simulink model "vegas01_4tap_1024ch_220_r7b"  (Simulink 7.6)
settings: Solver=ode45  StartTime=0.0  StopTime=128000  RelTol=1e-3
libraries: xbsIndex_r4 x13489, monroe_library x80, xps_library x51, casper_library_misc x43, xbsReplacements_r4 x42, dspsigops x16, simulink x7
(part 13 of 99)

== system vegas01_4tap_1024ch_220_r7b/pfb_fir_real3/coeff_gen_3_4 ==
  sync : Inport
  Counter0 : xbsIndex_r4/Counter  cnt_type=Free Running  cnt_to=Inf  operation=Up  start_count=8  cnt_by_val=1  arith_type=Unsigned  n_bits=8  bin_pt=0  load_pin=off  rst=on  en=off  explicit_period=on  period=1  dbl_ovrd=off  use_behavioral_HDL=off  implementation=Fabric  block_type=counter
  Counter1 : xbsIndex_r4/Counter  cnt_type=Free Running  cnt_to=Inf  operation=Down  start_count=247  cnt_by_val=1  arith_type=Unsigned  n_bits=8  bin_pt=0  load_pin=off  rst=on  en=off  explicit_period=on  period=1  dbl_ovrd=off  use_behavioral_HDL=off  implementation=Fabric  block_type=counter
  Delay16 : xbsIndex_r4/Delay  rst=off  infoeditControl=Selection of Reset will increase slice count due to use of real FFs and instead of SRLs  en=off  latency=2  dbl_ovrd=off  reg_retiming=off  block_type=delay
  Delay17 : xbsIndex_r4/Delay  rst=off  infoeditControl=Selection of Reset will increase slice count due to use of real FFs and instead of SRLs  en=off  latency=1  dbl_ovrd=off  reg_retiming=off  block_type=delay
  Delay18 : xbsIndex_r4/Delay  rst=off  infoeditControl=Selection of Reset will increase slice count due to use of real FFs and instead of SRLs  en=off  latency=1  dbl_ovrd=off  reg_retiming=off  block_type=delay
  Delay19 : xbsIndex_r4/Delay  rst=off  infoeditControl=Selection of Reset will increase slice count due to use of real FFs and instead of SRLs  en=off  latency=2  dbl_ovrd=off  reg_retiming=off  block_type=delay
  coeff_ram_1 : xbsIndex_r4/Dual Port RAM  depth=256  initVector=[-6.84185504899742e-05 -0.000225276188806986 -0.000382857821943778 -0.00054124611358964 -0.000700524248323099 -0.000860775882497438 -0.00102208509462138 -0.00118453633525111 -0.00134821437640948 -0.00151320426054887 -0.00167959124907416 -0.00184746077044269 -0.00201689836785779 -0.0021879896465735 -0.00236082022082727 -0.00253547566041853 -0.00271204143695053 -0.00289060286975338 -0.00307124507150638 -0.00325405289357752 -0.00343911087109885 -0.00362650316779578 -0.00381631352058928 -0.00400862518398944 -0.00420352087429949 -0.00440108271364903 -0.00460139217387592 -0.00480453002027584 -0.00501057625523885 -0.00521961006179269 -0.00543170974707191 -0.00564695268573309 -0.0058654152633352 -0.00608717281970556 -0.00631229959231075 -0.00654086865965289 -0.00677295188471086 -0.00700861985844697 -0.00724794184339896 -0.00749098571737739 -0.00773781791728902 -0.00798850338310597 -0.00824310550200131 -0.00850168605267101 -0.00876430514986291 -0.00903102118913259 -0.00930189079184692 -0.00957696875045512 -0.00985630797404788 -0.0101399594342249 -0.0104279721112905 -0.0107203929407984 -0.0110172667604652 -0.0113186362574725 -0.0116245419161785 -0.0119350219662582 -0.0122501123312931 -0.0125698465778293 -0.0128942558649243 -0.0132233688942021 -0.0135572118604367 -0.0138958084026824 -0.0142391795559714 -0.0145873437035975 -0.0149403165300052 -0.0152981109743031 -0.0156607371844211 -0.0160282024719291 -0.0164005112675375 -0.0167776650772961 -0.0171596624395113 -0.0175464988823994 -0.0179381668824935 -0.0183346558238221 -0.0187359519578779 -0.0191420383643933 -0.0195528949129399 -0.0199684982253701 -0.0203888216391169 -0.0208138351713685 -0.0212435054841348 -0.0216777958502221 -0.0221166661201311 -0.022560072689895 -0.0230079684698733 -0.0234603028545157 -0.0239170216931123 -0.0243780672615441 -0.0248433782350494 -0.0253128896620191 -0.0257865329388359 -0.0262642357857716 -0.0267459222239541 -0.02723151255342 -0.0277209233322634 -0.0282140673568951 -0.0287108536434236 -0.0292111874101705 -0.029714970061332 -0.0302220991717978 -0.0307324684731381 -0.0312459678407708 -0.0317624832823181 -0.0322818969271628 -0.0328040870172151 -0.0333289278988981 -0.0338562900163623 -0.0343860399059371 -0.0349180401918279 -0.0354521495830675 -0.0359882228717288 -0.0365261109324065 -0.0370656607229749 -0.0376067152866287 -0.038149113755212 -0.0386926913538438 -0.0392372794068422 -0.0397827053449559 -0.0403287927139051 -0.0408753611842385 -0.0414222265625081 -0.0419692008037677 -0.0425160920253971 -0.0430627045222547 -0.0436088387831623 -0.0441542915087227 -0.0446988556304727 -0.0452423203313728 -0.0457844710676339 -0.0463250895918826 -0.0468639539776635 -0.0474008386452801 -0.0479355143889729 -0.0484677484054324 -0.0489973043236477 -0.0495239422360871 -0.050047418731209 -0.0505674869273002 -0.0510838965076389 -0.0515963937569784 -0.0521047215993477 -0.0526086196371647 -0.0531078241916569 -0.0536020683445854 -0.0540910819812655 -0.0545745918348781 -0.0550523215320667 -0.0555239916398113 -0.0559893197135738 -0.0564480203467063 -0.0568998052211139 -0.0573443831591655 -0.0577814601768411 -0.0582107395381091 -0.0586319218105215 -0.0590447049220196 -0.059448784218937 -0.0598438525251917 -0.0602296002026545 -0.060605715212683 -0.0609718831788084 -0.0613277874505636 -0.0616731091684402 -0.0620075273299594 -0.0623307188568461 -0.0626423586632902 -0.0629421197252817 -0.0632296731510054 -0.0635046882522795 -0.0637668326170237 -0.0640157721827404 -0.0642511713109933 -0.0644726928628674 -0.0646799982753933 -0.0648727476389184 -0.0650505997754095 -0.0652132123176663 -0.0653602417894302 -0.0654913436863688 -0.0656061725579181 -0.0657043820899625 -0.0657856251883347 -0.0658495540631149 -0.0658958203137091 -0.0659240750146882 -0.0659339688023646 -0.0659251519620883 -0.0658972745162394 -0.0658499863128975 -0.0657829371151641 -0.0656957766911188 -0.0655881549043853 -0.0654597218052851 -0.0653101277225574 -0.0651390233556215 -0.0649460598673577 -0.0647308889773858 ...(+1181 chars)]  distributed_mem=Block RAM  init_a=0  init_b=0  rst_a=off  rst_b=off  en_a=off  en_b=off  latency=2  write_mode_A=No Read On Write  write_mode_B=No Read On Write  dbl_ovrd=off  optimize=Area  use_rpm=on  block_type=dpram
  coeff_ram_2 : xbsIndex_r4/Dual Port RAM  depth=256  initVector=[0.000926390151018473 0.00307160622155272 0.00525553833398869 0.00747830826159482 0.00974003139158092 0.0120408166550125 0.0143807664579271 0.0167599766136753 0.0191785362765084 0.0216365278764349 0.0241340270553677 0.0266711026045835 0.029247816403515 0.0318642233598974 0.0345203713512892 0.037216301167989 0.0399520464573652 0.0427276336696222 0.0455430820050192 0.0483984033625621 0.0512936022901863 0.0542286759364502 0.0572036140037557 0.0602183987031135 0.0632730047104714 0.066367399124622 0.0695015414267057 0.0726753834413258 0.0758888692992913 0.0791419354020027 0.0824345103874953 0.0857665150981551 0.0891378625501222 0.0925484579043931 0.0959981984396374 0.0994869735267415 0.103014664605091 0.106581145160602 0.110186280705523 0.113829928759999 0.117511938835429 0.121232152419619 0.124990402963731 0.128786515871061 0.132620308487624 0.136491590094586 0.140400161902524 0.144345817047543 0.148328340589239 0.152347509510534 0.156403092719369 0.160494851052275 0.164622537279825 0.168785896113961 0.172984664217222 0.17721857021385 0.181487334702801 0.18579067027265 0.190128281518395 0.194499865060165 0.198905109563833 0.203343695763531 0.20781529648607 0.212319576677266 0.216856193430172 0.221424796015206 0.226025025912192 0.230656516844288 0.235318894813825 0.240011778140024 0.244734777498616 0.249487495963348 0.254269529049357 0.259080464758442 0.263919883626188 0.268787358770969 0.273682455944801 0.27860473358605 0.283553742873988 0.288529027785176 0.293530125151685 0.298556564721129 0.303607869218511 0.308683554409866 0.313783129167691 0.318906095538162 0.324051948810107 0.32922017758574 0.334410263853137 0.339621683060439 0.344853904191773 0.350106389844876 0.355378596310409 0.36066997365294 0.36597996579359 0.37130801059432 0.376653539943846 0.382015979845165 0.387394750504671 0.392789266422851 0.398198936486543 0.403623164062721 0.409061347093818 0.414512878194538 0.419977144750163 0.425453529016308 0.430941408220135 0.43644015466298 0.441949135824379 0.447467714467479 0.452995248745804 0.458531092311357 0.464074594424031 0.469625100062317 0.475181950035267 0.480744481095709 0.486312026054677 0.491883913897031 0.497459469898254 0.503038015742378 0.508618869641048 0.51420134645366 0.519784757808577 0.525368412225376 0.530951615238117 0.536533669519589 0.54211387500652 0.547691529025715 0.553265926421101 0.558836359681638 0.564402119070086 0.569962492752582 0.575516766929012 0.581064225964134 0.586604152519439 0.592135827685706 0.597658531116231 0.603171541160694 0.608674134999635 0.614165588779514 0.619645177748309 0.625112176391646 0.6305658585694 0.636005497652767 0.641430366661742 0.646839738403009 0.652232885608173 0.657609081072333 0.662967597792947 0.668307709108963 0.673628688840178 0.678929811426806 0.684210352069207 0.68946958686775 0.694706792962786 0.699921248674682 0.705112233643903 0.710279028971086 0.7154209173571 0.720537183243032 0.725627112950088 0.730689994819365 0.735725119351461 0.740731779345898 0.745709270040315 0.750656889249406 0.755573937503563 0.760459718187202 0.765313537676731 0.770134705478122 0.774922534364069 0.779676340510692 0.784395443633751 0.789079167124339 0.793726838184032 0.798337787959446 0.802911351676187 0.807446868772144 0.811943683030113 0.816401142709704 0.820818600678503 0.825195414542469 0.829530946775514 0.833824564848259 0.838075641355911 0.842283554145252 0.846447686440694 0.850567426969378 0.854642170085277 0.858671315892297 0.862654270366307 0.866590445476112 0.870479259303306 0.874320136160995 0.87811250671135 0.881855808081977 0.885549483981054 0.889192984811226 0.892785767782227 0.896327297022184 0.899817043687609 0.903254486072019 0.906639109713177 0.909970407498925 0.913247879771583 0.916471034430877 0.919639387035394 0.922752460902521 0.925809787206842 0.928810905076992 0.931755361690913 0.934642712369514 0.937472520668701 0.940244358469748 0.942957806068012 0.945612452259933 0.948207894428338 0.950743738625992 0.953219599657401 0.955635101158839 ...(+645 chars)]  distributed_mem=Block RAM  init_a=0  init_b=0  rst_a=off  rst_b=off  en_a=off  en_b=off  latency=2  write_mode_A=No Read On Write  write_mode_B=No Read On Write  dbl_ovrd=off  optimize=Area  use_rpm=on  block_type=dpram
  coeff_ram_3 : xbsIndex_r4/Dual Port RAM  depth=256  initVector=[0.999993537908591 0.999930237468555 0.999799426219336 0.999601124319308 0.99933536232596 0.999002181189487 0.998601632244202 0.998133777197775 0.997598688118291 0.996996447419143 0.996327147841752 0.99559089243613 0.994787794539281 0.993917977751445 0.992981575910205 0.991978733062443 0.990909603434169 0.989774351398217 0.988573151439829 0.987306188120125 0.985973656037472 0.984575759786759 0.983112713916599 0.981584742884447 0.97999208100967 0.978334972424558 0.976613671023305 0.974828440408965 0.972979553838393 0.971067294165192 0.969091953780674 0.967053834552856 0.964953247763494 0.962790514043185 0.960565963304546 0.958279934673478 0.955932776418553 0.953524845878516 0.951056509387942 0.948528142201048 0.945940128413698 0.943292860883595 0.940586741148704 0.937822179343916 0.934999594115963 0.932119412536625 0.929182070014235 0.926188010203508 0.92313768491372 0.92003155401525 0.916870085344521 0.913653754607345 0.910383045280722 0.907058448513082 0.903680463023032 0.900249594996598 0.896766357983015 0.893231272789072 0.889644867372044 0.886007676731241 0.882320242798193 0.878583114325501 0.874796846774378 0.870962002200922 0.867079149141119 0.863148862494636 0.859171723407407 0.855148319153063 0.851079243013208 0.846965094156595 0.842806477517219 0.83860400367135 0.834358288713558 0.830069954131732 0.825739626681142 0.821367938257571 0.81695552576954 0.812503031009668 0.808011100525185 0.803480385487643 0.798911541561843 0.794305228774019 0.789662111379308 0.78498285772853 0.780268140134329 0.775518634736677 0.77073502136781 0.765917983416591 0.761068207692364 0.756186384288308 0.751273206444335 0.746329370409569 0.74135557530442 0.736352522982309 0.731320917891057 0.726261466933986 0.721174879330752 0.716061866477951 0.710923141809532 0.705759420657041 0.700571420109737 0.695359858874611 0.690125457136339 0.6848689364172 0.679591019437003 0.674292429973034 0.668973892720087 0.663636133150573 0.658279877374778 0.652905852001274 0.647514783997532 0.642107400550756 0.636684428928989 0.631246596342501 0.625794629805507 0.620329255998239 0.614851201129411 0.609361190799097 0.603859949862066 0.598348202291596 0.592826671043806 0.587296077922522 0.581757143444736 0.576210586706653 0.570657125250384 0.565097474931304 0.559532349786106 0.553962461901578 0.548388521284143 0.542811235730172 0.537231310697124 0.53164944917552 0.526066351561788 0.520482715532013 0.51489923591661 0.509316604575952 0.503735510276982 0.498156638570832 0.492580671671482 0.487008288335481 0.48144016374275 0.475876969378513 0.470319372916355 0.464768038102454 0.459223624641001 0.453686788080837 0.448158179703323 0.44263844641148 0.437128230620413 0.431628170149044 0.426138898113178 0.420661042819923 0.415195227663486 0.409742071022372 0.404302186157994 0.398876181114731 0.393464658621442 0.388068215994463 0.382687445042102 0.377322931970653 0.371975257291953 0.366644995732486 0.361332716144072 0.356038981416135 0.350764348389594 0.345509367772363 0.340274584056508 0.335060535437048 0.329867753732433 0.324696764306712 0.319548085993394 0.314422231021032 0.309319704940528 0.304241006554183 0.299186627846502 0.294157053916756 0.289152762913333 0.284174225969862 0.279221907143152 0.274296263352921 0.269397744323361 0.264526792526511 0.259683843127481 0.254869323931514 0.250083655332892 0.245327250265711 0.240600514156511 0.235903844878785 0.231237632709359 0.22660226028665 0.221998102570821 0.217425526805815 0.212884892483285 0.208376551308429 0.20390084716771 0.199458116098491 0.195048686260562 0.190672877909583 0.186331003372418 0.182023367024387 0.177750265268411 0.173511986516071 0.169308811170563 0.165141011611557 0.161008852181946 0.156912589176507 0.152852470832436 0.148828737321782 0.144841620745766 0.140891345130968 0.136978126427406 0.133102172508466 0.129263683172704 0.125462850147503 0.121699857094577 0.117974879617314 0.114288085269957 0.110639633568606 0.107029676004035 0.103458356056317 0.0999258092112481 0.0964321629785505 0.092977536911857 ...(+625 chars)]  distributed_mem=Block RAM  init_a=0  init_b=0  rst_a=off  rst_b=off  en_a=off  en_b=off  latency=2  write_mode_A=No Read On Write  write_mode_B=No Read On Write  dbl_ovrd=off  optimize=Area  use_rpm=on  block_type=dpram
  coeff_ram_4 : xbsIndex_r4/Dual Port RAM  depth=256  initVector=[-0.000919015198046551 -0.00299198883650501 -0.00502663145358993 -0.00702308888506142 -0.0089815129946132 -0.0109020615971933 -0.0127848983812844 -0.0146301928301678 -0.0164381201421957 -0.0182088611500971 -0.0199426022393407 -0.0216395352655827 -0.023299857471221 -0.0249237714010852 -0.0265114848172841 -0.0280632106132407 -0.029579166726936 -0.0310595760533919 -0.0325046663564158 -0.0339146701796359 -0.0352898247568515 -0.0366303719217263 -0.0379365580168502 -0.039208633802197 -0.0404468543630039 -0.0416514790171007 -0.0428227712217146 -0.043960998479778 -0.045066432245766 -0.046139347831091 -0.0471800243090809 -0.0481887444195689 -0.0491657944731203 -0.0501114642549257 -0.0510260469283856 -0.0519098389384146 -0.052763139914492 -0.0535862525734862 -0.0543794826222791 -0.0551431386602185 -0.0558775320814249 -0.0565829769769793 -0.05725979003702 -0.0579082904527735 -0.0585287998185482 -0.0591216420337153 -0.0596871432047058 -0.0602256315470477 -0.060737437287472 -0.0612228925661118 -0.0616823313388219 -0.0621160892796445 -0.0625245036834464 -0.0629079133687547 -0.0632666585808146 -0.0636010808948972 -0.0639115231198798 -0.064198329202127 -0.0644618441296943 -0.0647024138368813 -0.0649203851091579 -0.0651161054884889 -0.0652899231790798 -0.0654421869535685 -0.0655732460596878 -0.0656834501274199 -0.0657731490766683 -0.0658426930254688 -0.0658924321987641 -0.0659227168377624 -0.0659338971099046 -0.0659263230194608 -0.0659003443187784 -0.0658563104202036 -0.0657945703086977 -0.0657154724551687 -0.0656193647305391 -0.0655065943205716 -0.0653775076414712 -0.0652324502562844 -0.0650717667921157 -0.0648958008581796 -0.0647048949647076 -0.0644993904427296 -0.0642796273647465 -0.0640459444663137 -0.063798679068552 -0.0635381670016044 -0.0632647425290539 -0.0629787382733223 -0.0626804851420626 -0.062370312255564 -0.0620485468751832 -0.0617155143328191 -0.0613715379614439 -0.0610169390267068 -0.0606520366596237 -0.060277147790367 -0.0598925870831692 -0.0594986668723528 -0.0590956970995 -0.058683985251774 -0.0582638363014045 -0.0578355526463479 -0.0573994340521345 -0.0569557775949133 -0.0565048776057046 -0.0560470256158701 -0.0555825103038107 -0.0551116174429017 -0.0546346298506727 -0.0541518273392423 -0.0536634866670147 -0.0531698814916457 -0.0526712823242868 -0.0521679564851118 -0.0516601680601348 -0.0511481778593241 -0.0506322433760179 -0.0501126187476476 -0.049589554717773 -0.0490632985994332 -0.0485340942398192 -0.0480021819862691 -0.0474677986535914 -0.0469311774927178 -0.0463925481606885 -0.0458521366919714 -0.0453101654711176 -0.0447668532067534 -0.04422241490691 -0.0436770618556918 -0.0431310015912819 -0.0425844378852857 -0.0420375707234105 -0.0414905962874812 -0.0409437069387892 -0.0403970912027733 -0.0398509337550292 -0.0393054154086462 -0.0387607131028666 -0.0382169998930642 -0.0376744449420393 -0.0371332135126239 -0.036593466961594 -0.0360553627348824 -0.0355190543640875 -0.0349846914642725 -0.0344524197330471 -0.0339223809509266 -0.0333947129829612 -0.0328695497816274 -0.0323470213909745 -0.0318272539520174 -0.0313103697093688 -0.0307964870190998 -0.0302857203578219 -0.0297781803329794 -0.0292739736943434 -0.0287732033466972 -0.0282759683637013 -0.0277823640029301 -0.0272924817220655 -0.0268064091962394 -0.0263242303365116 -0.0258460253094707 -0.025371870557947 -0.0249018388228245 -0.0244359991659379 -0.0239744169940429 -0.0235171540838455 -0.0230642686080765 -0.0226158151625978 -0.0221718447945254 -0.0217324050313552 -0.0212975399110761 -0.0208672900132567 -0.0204416924910876 -0.0200207811043675 -0.0196045862534134 -0.0191931350138819 -0.0187864511724837 -0.0183845552635757 -0.0179874646066126 -0.0175951933444434 -0.0172077524824336 -0.016825149928397 -0.0164473905333187 -0.0160744761328528 -0.0157064055895765 -0.0153431748359811 -0.0149847769181846 -0.0146312020403452 -0.0142824376097579 -0.013938468282616 -0.0135992760104181 -0.0132648400870021 -0.0129351371961867 -0.0126101414600018 -0.0122898244874881 ...(+1170 chars)]  distributed_mem=Block RAM  init_a=0  init_b=0  rst_a=off  rst_b=off  en_a=off  en_b=off  latency=2  write_mode_A=No Read On Write  write_mode_B=No Read On Write  dbl_ovrd=off  optimize=Area  use_rpm=on  block_type=dpram
  const_din : xbsIndex_r4/Constant  const=0  gui_display_data_type=Fixed-point  arith_type=Signed (2's comp)  n_bits=18  bin_pt=17  preci_type=Single  exp_width=8  frac_width=24  explicit_period=on  period=1  equ=P=C  opselect=C  inp2=PCIN>>17  opr=+  inp1=P  carry=CIN  dbl_ovrd=off  block_type=constant
  const_we : xbsIndex_r4/Constant  const=0  gui_display_data_type=Fixed-point  arith_type=Boolean  n_bits=16  bin_pt=14  preci_type=Single  exp_width=8  frac_width=24  explicit_period=on  period=1  equ=P=C  opselect=C  inp2=PCIN>>17  opr=+  inp1=P  carry=CIN  dbl_ovrd=off  block_type=constant
  cram_lower : Subsystem
  cram_upper : Subsystem
  sync_out : Outport
  coeff : Outport  Port=2
  coeff_rev : Outport  Port=3
  wires:
  sync/1 -> Counter1/1, Counter0/1, sync_out/1
  Counter0/1 -> coeff_ram_4/1, coeff_ram_3/1, Delay17/1, Delay16/1
  const_din/1 -> coeff_ram_4/5, coeff_ram_4/2, coeff_ram_3/5, coeff_ram_3/2, coeff_ram_2/5, coeff_ram_2/2, coeff_ram_1/5, coeff_ram_1/2
  const_we/1 -> coeff_ram_4/6, coeff_ram_4/3, coeff_ram_3/6, coeff_ram_3/3, coeff_ram_2/6, coeff_ram_2/3, coeff_ram_1/6, coeff_ram_1/3
  Counter1/1 -> Delay19/1, Delay18/1, coeff_ram_2/4, coeff_ram_1/4
  cram_lower/1(out) -> coeff_rev/1
  cram_upper/1(out) -> coeff/1
  coeff_ram_1/2 -> cram_lower/4(in3)
  coeff_ram_2/2 -> cram_lower/3(in2)
  coeff_ram_3/2 -> cram_lower/2(in1)
  coeff_ram_4/2 -> cram_lower/1(in0)
  coeff_ram_4/1 -> cram_upper/4(in3)
  coeff_ram_3/1 -> cram_upper/3(in2)
  coeff_ram_2/1 -> cram_upper/2(in1)
  coeff_ram_1/1 -> cram_upper/1(in0)
  Delay19/1 -> coeff_ram_4/4
  Delay18/1 -> coeff_ram_3/4
  Delay17/1 -> coeff_ram_2/1
  Delay16/1 -> coeff_ram_1/1

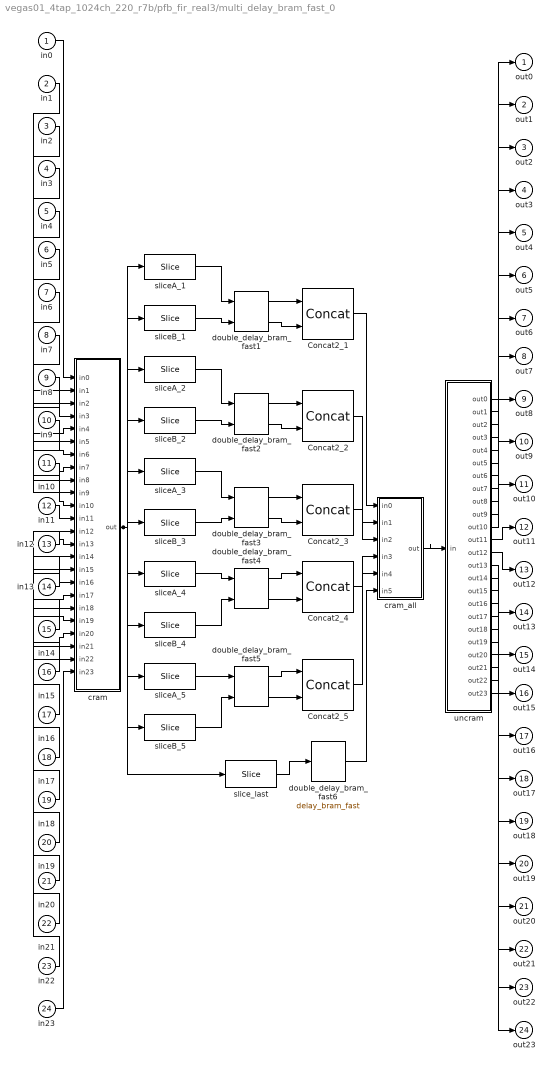
== system vegas01_4tap_1024ch_220_r7b/pfb_fir_real3/multi_delay_bram_fast_0 ==
  in0 : Inport
  in1 : Inport  Port=2
  in2 : Inport  Port=3
  in3 : Inport  Port=4
  in4 : Inport  Port=5
  in5 : Inport  Port=6
  in6 : Inport  Port=7
  in7 : Inport  Port=8
  in8 : Inport  Port=9
  in9 : Inport  Port=10
  in10 : Inport  Port=11
  in11 : Inport  Port=12
  in12 : Inport  Port=13
  in13 : Inport  Port=14
  in14 : Inport  Port=15
  in15 : Inport  Port=16
  in16 : Inport  Port=17
  in17 : Inport  Port=18
  in18 : Inport  Port=19
  in19 : Inport  Port=20
  in20 : Inport  Port=21
  in21 : Inport  Port=22
  in22 : Inport  Port=23
  in23 : Inport  Port=24
  Concat2_{1..5} (5 alike) : xbsIndex_r4/Concat  num_inputs=2  dbl_ovrd=off  block_type=concat
  cram : Subsystem
  cram_all : Subsystem
  double_delay_bram_fast{1..5} (5 alike) : monroe_library/double_delay_bram_fast  SystemSampleTime=-1  delay_len=256  bram_latency=2  register_counter=1
  double_delay_bram_fast6 : monroe_library/delay_bram_fast  SystemSampleTime=-1  delay_len=256  bram_latency=2  memory_type=Block RAM  register_counter=1
  sliceA_1 : xbsIndex_r4/Slice  nbits=18  boolean_output=off  mode=Upper Bit Location + Width  bit1=-0  base1=MSB of Input  bit0=-0  base0=MSB of Input  dbl_ovrd=off  block_type=slice
  sliceA_2 : xbsIndex_r4/Slice  nbits=18  boolean_output=off  mode=Upper Bit Location + Width  bit1=-36  base1=MSB of Input  bit0=-36  base0=MSB of Input  dbl_ovrd=off  block_type=slice
  sliceA_3 : xbsIndex_r4/Slice  nbits=18  boolean_output=off  mode=Upper Bit Location + Width  bit1=-72  base1=MSB of Input  bit0=-72  base0=MSB of Input  dbl_ovrd=off  block_type=slice
  sliceA_4 : xbsIndex_r4/Slice  nbits=18  boolean_output=off  mode=Upper Bit Location + Width  bit1=-108  base1=MSB of Input  bit0=-108  base0=MSB of Input  dbl_ovrd=off  block_type=slice
  sliceA_5 : xbsIndex_r4/Slice  nbits=18  boolean_output=off  mode=Upper Bit Location + Width  bit1=-144  base1=MSB of Input  bit0=-144  base0=MSB of Input  dbl_ovrd=off  block_type=slice
  sliceB_1 : xbsIndex_r4/Slice  nbits=18  boolean_output=off  mode=Upper Bit Location + Width  bit1=-18  base1=MSB of Input  bit0=-18  base0=MSB of Input  dbl_ovrd=off  block_type=slice
  sliceB_2 : xbsIndex_r4/Slice  nbits=18  boolean_output=off  mode=Upper Bit Location + Width  bit1=-54  base1=MSB of Input  bit0=-54  base0=MSB of Input  dbl_ovrd=off  block_type=slice
  sliceB_3 : xbsIndex_r4/Slice  nbits=18  boolean_output=off  mode=Upper Bit Location + Width  bit1=-90  base1=MSB of Input  bit0=-90  base0=MSB of Input  dbl_ovrd=off  block_type=slice
  sliceB_4 : xbsIndex_r4/Slice  nbits=18  boolean_output=off  mode=Upper Bit Location + Width  bit1=-126  base1=MSB of Input  bit0=-126  base0=MSB of Input  dbl_ovrd=off  block_type=slice
  sliceB_5 : xbsIndex_r4/Slice  nbits=18  boolean_output=off  mode=Upper Bit Location + Width  bit1=-162  base1=MSB of Input  bit0=-162  base0=MSB of Input  dbl_ovrd=off  block_type=slice
  slice_last : xbsIndex_r4/Slice  nbits=12  boolean_output=off  mode=Lower Bit Location + Width  bit1=0  base1=LSB of Input  bit0=0  base0=LSB of Input  dbl_ovrd=off  block_type=slice
  uncram : Subsystem
  out0 : Outport
  out1 : Outport  Port=2
  out2 : Outport  Port=3
  out3 : Outport  Port=4
  out4 : Outport  Port=5
  out5 : Outport  Port=6
  out6 : Outport  Port=7
  out7 : Outport  Port=8
  out8 : Outport  Port=9
  out9 : Outport  Port=10
  out10 : Outport  Port=11
  out11 : Outport  Port=12
  out12 : Outport  Port=13
  out13 : Outport  Port=14
  out14 : Outport  Port=15
  out15 : Outport  Port=16
  out16 : Outport  Port=17
  out17 : Outport  Port=18
  out18 : Outport  Port=19
  out19 : Outport  Port=20
  out20 : Outport  Port=21
  out21 : Outport  Port=22
  out22 : Outport  Port=23
  out23 : Outport  Port=24
  wires:
  cram/1(out) -> slice_last/1, sliceB_5/1, sliceA_5/1, sliceB_4/1, sliceA_4/1, sliceB_3/1, sliceA_3/1, sliceB_2/1, sliceA_2/1, sliceB_1/1, sliceA_1/1
  uncram/24(out23) -> out23/1
  uncram/23(out22) -> out22/1
  uncram/22(out21) -> out21/1
  uncram/21(out20) -> out20/1
  uncram/20(out19) -> out19/1
  uncram/19(out18) -> out18/1
  uncram/18(out17) -> out17/1
  uncram/17(out16) -> out16/1
  uncram/16(out15) -> out15/1
  uncram/15(out14) -> out14/1
  uncram/14(out13) -> out13/1
  uncram/13(out12) -> out12/1
  uncram/12(out11) -> out11/1
  uncram/11(out10) -> out10/1
  uncram/10(out9) -> out9/1
  uncram/9(out8) -> out8/1
  uncram/8(out7) -> out7/1
  uncram/7(out6) -> out6/1
  uncram/6(out5) -> out5/1
  uncram/5(out4) -> out4/1
  uncram/4(out3) -> out3/1
  uncram/3(out2) -> out2/1
  uncram/2(out1) -> out1/1
  uncram/1(out0) -> out0/1
  cram_all/1(out) -> uncram/1(in)
  double_delay_bram_fast6/1 -> cram_all/6(in5)
  Concat2_5/1 -> cram_all/5(in4)
  Concat2_4/1 -> cram_all/4(in3)
  Concat2_3/1 -> cram_all/3(in2)
  Concat2_2/1 -> cram_all/2(in1)
  Concat2_1/1 -> cram_all/1(in0)
  slice_last/1 -> double_delay_bram_fast6/1
  double_delay_bram_fast5/2 -> Concat2_5/2
  double_delay_bram_fast5/1 -> Concat2_5/1
  sliceB_5/1 -> double_delay_bram_fast5/2
  sliceA_5/1 -> double_delay_bram_fast5/1
  double_delay_bram_fast4/2 -> Concat2_4/2
  double_delay_bram_fast4/1 -> Concat2_4/1
  sliceB_4/1 -> double_delay_bram_fast4/2
  sliceA_4/1 -> double_delay_bram_fast4/1
  double_delay_bram_fast3/2 -> Concat2_3/2
  double_delay_bram_fast3/1 -> Concat2_3/1
  sliceB_3/1 -> double_delay_bram_fast3/2
  sliceA_3/1 -> double_delay_bram_fast3/1
  double_delay_bram_fast2/2 -> Concat2_2/2
  double_delay_bram_fast2/1 -> Concat2_2/1
  sliceB_2/1 -> double_delay_bram_fast2/2
  sliceA_2/1 -> double_delay_bram_fast2/1
  double_delay_bram_fast1/2 -> Concat2_1/2
  double_delay_bram_fast1/1 -> Concat2_1/1
  sliceB_1/1 -> double_delay_bram_fast1/2
  sliceA_1/1 -> double_delay_bram_fast1/1
  in23/1 -> cram/24(in23)
  in22/1 -> cram/23(in22)
  in21/1 -> cram/22(in21)
  in20/1 -> cram/21(in20)
  in19/1 -> cram/20(in19)
  in18/1 -> cram/19(in18)
  in17/1 -> cram/18(in17)
  in16/1 -> cram/17(in16)
  in15/1 -> cram/16(in15)
  in14/1 -> cram/15(in14)
  in13/1 -> cram/14(in13)
  in12/1 -> cram/13(in12)
  in11/1 -> cram/12(in11)
  in10/1 -> cram/11(in10)
  in9/1 -> cram/10(in9)
  in8/1 -> cram/9(in8)
  in7/1 -> cram/8(in7)
  in6/1 -> cram/7(in6)
  in5/1 -> cram/6(in5)
  in4/1 -> cram/5(in4)
  in3/1 -> cram/4(in3)
  in2/1 -> cram/3(in2)
  in1/1 -> cram/2(in1)
  in0/1 -> cram/1(in0)

== system vegas01_4tap_1024ch_220_r7b/pktsnap/bram_lsb ==
  addr : Inport
  data_in : Inport  Port=2
  we : Inport  Port=3
  calc_add : Subsystem
  convert_addr : xbsIndex_r4/Convert  gui_display_data_type=Fixed-point  arith_type=Unsigned  n_bits=addr_width  bin_pt=0  float_type=Single  exp_bits=8  fraction_bits=24  quantization=Truncate  overflow=Wrap  en=off  latency=0  dbl_ovrd=off  pipeline=off  block_type=convert
      settings: xl_area=[0, 0, 0, 0, 0, 0, 0]
  convert_din : xbsIndex_r4/Convert  gui_display_data_type=Fixed-point  arith_type=Unsigned  n_bits=data_width  bin_pt=data_bin_pt  float_type=Single  exp_bits=8  fraction_bits=24  quantization=Truncate  overflow=Wrap  en=off  latency=0  dbl_ovrd=off  pipeline=off  block_type=convert
      settings: xl_area=[0, 0, 0, 0, 0, 0, 0]
  convert_din1 : xbsIndex_r4/Convert  gui_display_data_type=Fixed-point  arith_type=Unsigned  n_bits=data_width  bin_pt=data_bin_pt  float_type=Single  exp_bits=8  fraction_bits=24  quantization=Truncate  overflow=Wrap  en=off  latency=0  dbl_ovrd=off  pipeline=off  block_type=convert
      settings: xl_area=[0, 0, 0, 0, 0, 0, 0]
  convert_we : xbsIndex_r4/Convert  gui_display_data_type=Fixed-point  arith_type=Unsigned  n_bits=1  bin_pt=0  float_type=Single  exp_bits=8  fraction_bits=24  quantization=Truncate  overflow=Wrap  en=off  latency=0  dbl_ovrd=off  pipeline=off  block_type=convert
      settings: xl_area=[0, 0, 0, 0, 0, 0, 0]
  mem : Subsystem
  munge_in : Subsystem  AncestorBlock=casper_library_flow_control/munge  mask(munge){divisions=1, div_size=32, order=[0]}
      mask prompts: divisions "Number of divisions"; div_size "Division size (bits)"; order "Division packing order"
      mask help: Splits an input bus into the specified number of divisions and then recombines them in the order specified (most significant division = 0,least = number divisions-1)
      mask init:
      | munge_init(gcb, ...
      |     'divisions', divisions, ...
      |     'div_size', div_size, ...
      |     'order', order);
      settings: AttributesFormatString=|
      | [0]
      | 32 bits
  munge_out : Subsystem  AncestorBlock=casper_library_flow_control/munge  mask(munge){divisions=1, div_size=32, order=[0]}
      mask prompts: divisions "Number of divisions"; div_size "Division size (bits)"; order "Division packing order"
      mask help: Splits an input bus into the specified number of divisions and then recombines them in the order specified (most significant division = 0,least = number divisions-1)
      mask init:
      | munge_init(gcb, ...
      |     'divisions', divisions, ...
      |     'div_size', div_size, ...
      |     'order', order);
      settings: AttributesFormatString=|
      | [0]
      | 32 bits
  vegas01_4tap_1024ch_220_r6i_pktsnap_bram_lsb_addr : xbsIndex_r4/Gateway Out  hdl_port=on  timing_constraint=None  locs_specified=off  LOCs={'A5','B9','A9','C1','A3','G6','E9','A6','A7','A8','B8','B1','B3','D9','B5','B4','B6','C2'}  block_type=gatewayout
      settings: infoedit="Gateway out block.  Converts Xilinx fixed point inputs into ouputs of type Simulink integer, double, or fixed point.<P><P>Hardware notes:  In hardware these blocks become top level output ports or are discarded, depending on how they are configured."  xl_area=[0, 0, 0, 0, 0, 0, 0]  sginterface={'Nallatech'=>{'xdspkit'=>{'non_memory_mapped_port'=>'uprev_Shared_BRAM_addr'}},'Xilinx'=>{'jtaghwcosim'=>{'non_memory_mapped_port'=>'uprev_Shared_BRAM_addr'},'xdspkit'=>{'non_memory_mapped_port'=>'uprev_Shared_BRAM_addr'}}}
  vegas01_4tap_1024ch_220_r6i_pktsnap_bram_lsb_data_in : xbsIndex_r4/Gateway Out  hdl_port=on  timing_constraint=None  locs_specified=off  LOCs={'B21','B22','A22','B23','B19','C19','A20','A21','A23','B24','A24','A25','E18','D18','C18','B18'}  block_type=gatewayout
      settings: infoedit="Gateway out block.  Converts Xilinx fixed point inputs into ouputs of type Simulink integer, double, or fixed point.<P><P>Hardware notes:  In hardware these blocks become top level output ports or are discarded, depending on how they are configured."  xl_area=[0, 0, 0, 0, 0, 0, 0]  sginterface={'Nallatech'=>{'xdspkit'=>{'non_memory_mapped_port'=>'uprev_Shared_BRAM_data_in'}},'Xilinx'=>{'jtaghwcosim'=>{'non_memory_mapped_port'=>'uprev_Shared_BRAM_data_in'},'xdspkit'=>{'non_memory_mapped_port'=>'uprev_Shared_BRAM_data_in'}}}
  vegas01_4tap_1024ch_220_r6i_pktsnap_bram_lsb_data_out : xbsIndex_r4/Gateway In  gui_display_data_type=Fixed-point  arith_type=Unsigned  n_bits=data_width  bin_pt=data_bin_pt  preci_type=Single  exp_width=8  frac_width=24  quantization=Truncate  overflow=Wrap  period=sample_rate  dbl_ovrd=off  timing_constraint=None  locs_specified=off  LOCs={}  hdl_port=on  block_type=gatewayin
      settings: infoedit="Gateway in block.  Converts inputs of type Simulink integer, double and fixed point to  Xilinx fixed point type.<br><br>Hardware notes:  In hardware these blocks become top level input ports."  xl_area=[0, 0, 0, 0, 0, 0, 0]
  vegas01_4tap_1024ch_220_r6i_pktsnap_bram_lsb_we : xbsIndex_r4/Gateway Out  hdl_port=on  timing_constraint=None  locs_specified=off  LOCs={'A14'}  block_type=gatewayout
      settings: infoedit="Gateway out block.  Converts Xilinx fixed point inputs into ouputs of type Simulink integer, double, or fixed point.<P><P>Hardware notes:  In hardware these blocks become top level output ports or are discarded, depending on how they are configured."  xl_area=[0, 0, 0, 0, 0, 0, 0]
  data_out : Outport
  wires:
  convert_din/1 -> munge_in/1(din)
  convert_din1/1 -> vegas01_4tap_1024ch_220_r6i_pktsnap_bram_lsb_data_in/1
  convert_addr/1 -> vegas01_4tap_1024ch_220_r6i_pktsnap_bram_lsb_addr/1
  calc_add/1(out) -> convert_addr/1
  munge_out/1(dout) -> data_out/1
  munge_in/1(dout) -> convert_din1/1
  vegas01_4tap_1024ch_220_r6i_pktsnap_bram_lsb_we/1 -> mem/3(In3)
  vegas01_4tap_1024ch_220_r6i_pktsnap_bram_lsb_data_in/1 -> mem/2(In2)
  vegas01_4tap_1024ch_220_r6i_pktsnap_bram_lsb_addr/1 -> mem/1(In1)
  convert_we/1 -> vegas01_4tap_1024ch_220_r6i_pktsnap_bram_lsb_we/1
  we/1 -> convert_we/1
  addr/1 -> calc_add/1(in)
  vegas01_4tap_1024ch_220_r6i_pktsnap_bram_lsb_data_out/1 -> munge_out/1(din)
  data_in/1 -> convert_din/1
  mem/1(Out1) -> vegas01_4tap_1024ch_220_r6i_pktsnap_bram_lsb_data_out/1

== system vegas01_4tap_1024ch_220_r7b/pktsnap/bram_msb ==
  addr : Inport
  data_in : Inport  Port=2
  we : Inport  Port=3
  calc_add : Subsystem
  convert_addr : xbsIndex_r4/Convert  gui_display_data_type=Fixed-point  arith_type=Unsigned  n_bits=addr_width  bin_pt=0  float_type=Single  exp_bits=8  fraction_bits=24  quantization=Truncate  overflow=Wrap  en=off  latency=0  dbl_ovrd=off  pipeline=off  block_type=convert
      settings: xl_area=[0, 0, 0, 0, 0, 0, 0]
  convert_din : xbsIndex_r4/Convert  gui_display_data_type=Fixed-point  arith_type=Unsigned  n_bits=data_width  bin_pt=data_bin_pt  float_type=Single  exp_bits=8  fraction_bits=24  quantization=Truncate  overflow=Wrap  en=off  latency=0  dbl_ovrd=off  pipeline=off  block_type=convert
      settings: xl_area=[0, 0, 0, 0, 0, 0, 0]
  convert_din1 : xbsIndex_r4/Convert  gui_display_data_type=Fixed-point  arith_type=Unsigned  n_bits=data_width  bin_pt=data_bin_pt  float_type=Single  exp_bits=8  fraction_bits=24  quantization=Truncate  overflow=Wrap  en=off  latency=0  dbl_ovrd=off  pipeline=off  block_type=convert
      settings: xl_area=[0, 0, 0, 0, 0, 0, 0]
  convert_we : xbsIndex_r4/Convert  gui_display_data_type=Fixed-point  arith_type=Unsigned  n_bits=1  bin_pt=0  float_type=Single  exp_bits=8  fraction_bits=24  quantization=Truncate  overflow=Wrap  en=off  latency=0  dbl_ovrd=off  pipeline=off  block_type=convert
      settings: xl_area=[0, 0, 0, 0, 0, 0, 0]
  mem : Subsystem
  munge_in : Subsystem  AncestorBlock=casper_library_flow_control/munge  mask(munge){divisions=1, div_size=32, order=[0]}
      mask prompts: divisions "Number of divisions"; div_size "Division size (bits)"; order "Division packing order"
      mask help: Splits an input bus into the specified number of divisions and then recombines them in the order specified (most significant division = 0,least = number divisions-1)
      mask init:
      | munge_init(gcb, ...
      |     'divisions', divisions, ...
      |     'div_size', div_size, ...
      |     'order', order);
      settings: AttributesFormatString=|
      | [0]
      | 32 bits
  munge_out : Subsystem  AncestorBlock=casper_library_flow_control/munge  mask(munge){divisions=1, div_size=32, order=[0]}
      mask prompts: divisions "Number of divisions"; div_size "Division size (bits)"; order "Division packing order"
      mask help: Splits an input bus into the specified number of divisions and then recombines them in the order specified (most significant division = 0,least = number divisions-1)
      mask init:
      | munge_init(gcb, ...
      |     'divisions', divisions, ...
      |     'div_size', div_size, ...
      |     'order', order);
      settings: AttributesFormatString=|
      | [0]
      | 32 bits
  vegas01_4tap_1024ch_220_r6i_pktsnap_bram_msb_addr : xbsIndex_r4/Gateway Out  hdl_port=on  timing_constraint=None  locs_specified=off  LOCs={'A5','B9','A9','C1','A3','G6','E9','A6','A7','A8','B8','B1','B3','D9','B5','B4','B6','C2'}  block_type=gatewayout
      settings: infoedit="Gateway out block.  Converts Xilinx fixed point inputs into ouputs of type Simulink integer, double, or fixed point.<P><P>Hardware notes:  In hardware these blocks become top level output ports or are discarded, depending on how they are configured."  xl_area=[0, 0, 0, 0, 0, 0, 0]  sginterface={'Nallatech'=>{'xdspkit'=>{'non_memory_mapped_port'=>'uprev_Shared_BRAM_addr'}},'Xilinx'=>{'jtaghwcosim'=>{'non_memory_mapped_port'=>'uprev_Shared_BRAM_addr'},'xdspkit'=>{'non_memory_mapped_port'=>'uprev_Shared_BRAM_addr'}}}
  vegas01_4tap_1024ch_220_r6i_pktsnap_bram_msb_data_in : xbsIndex_r4/Gateway Out  hdl_port=on  timing_constraint=None  locs_specified=off  LOCs={'B21','B22','A22','B23','B19','C19','A20','A21','A23','B24','A24','A25','E18','D18','C18','B18'}  block_type=gatewayout
      settings: infoedit="Gateway out block.  Converts Xilinx fixed point inputs into ouputs of type Simulink integer, double, or fixed point.<P><P>Hardware notes:  In hardware these blocks become top level output ports or are discarded, depending on how they are configured."  xl_area=[0, 0, 0, 0, 0, 0, 0]  sginterface={'Nallatech'=>{'xdspkit'=>{'non_memory_mapped_port'=>'uprev_Shared_BRAM_data_in'}},'Xilinx'=>{'jtaghwcosim'=>{'non_memory_mapped_port'=>'uprev_Shared_BRAM_data_in'},'xdspkit'=>{'non_memory_mapped_port'=>'uprev_Shared_BRAM_data_in'}}}
  vegas01_4tap_1024ch_220_r6i_pktsnap_bram_msb_data_out : xbsIndex_r4/Gateway In  gui_display_data_type=Fixed-point  arith_type=Unsigned  n_bits=data_width  bin_pt=data_bin_pt  preci_type=Single  exp_width=8  frac_width=24  quantization=Truncate  overflow=Wrap  period=sample_rate  dbl_ovrd=off  timing_constraint=None  locs_specified=off  LOCs={}  hdl_port=on  block_type=gatewayin
      settings: infoedit="Gateway in block.  Converts inputs of type Simulink integer, double and fixed point to  Xilinx fixed point type.<br><br>Hardware notes:  In hardware these blocks become top level input ports."  xl_area=[0, 0, 0, 0, 0, 0, 0]
  vegas01_4tap_1024ch_220_r6i_pktsnap_bram_msb_we : xbsIndex_r4/Gateway Out  hdl_port=on  timing_constraint=None  locs_specified=off  LOCs={'A14'}  block_type=gatewayout
      settings: infoedit="Gateway out block.  Converts Xilinx fixed point inputs into ouputs of type Simulink integer, double, or fixed point.<P><P>Hardware notes:  In hardware these blocks become top level output ports or are discarded, depending on how they are configured."  xl_area=[0, 0, 0, 0, 0, 0, 0]
  data_out : Outport
  wires:
  convert_din/1 -> munge_in/1(din)
  convert_din1/1 -> vegas01_4tap_1024ch_220_r6i_pktsnap_bram_msb_data_in/1
  convert_addr/1 -> vegas01_4tap_1024ch_220_r6i_pktsnap_bram_msb_addr/1
  calc_add/1(out) -> convert_addr/1
  munge_out/1(dout) -> data_out/1
  munge_in/1(dout) -> convert_din1/1
  vegas01_4tap_1024ch_220_r6i_pktsnap_bram_msb_we/1 -> mem/3(In3)
  vegas01_4tap_1024ch_220_r6i_pktsnap_bram_msb_data_in/1 -> mem/2(In2)
  vegas01_4tap_1024ch_220_r6i_pktsnap_bram_msb_addr/1 -> mem/1(In1)
  convert_we/1 -> vegas01_4tap_1024ch_220_r6i_pktsnap_bram_msb_we/1
  we/1 -> convert_we/1
  addr/1 -> calc_add/1(in)
  vegas01_4tap_1024ch_220_r6i_pktsnap_bram_msb_data_out/1 -> munge_out/1(din)
  data_in/1 -> convert_din/1
  mem/1(Out1) -> vegas01_4tap_1024ch_220_r6i_pktsnap_bram_msb_data_out/1

== system vegas01_4tap_1024ch_220_r7b/sg/bus_expand ==
  bus_in : Inport
  reinterpret{1..4} (4 alike) : xbsIndex_r4/Reinterpret  force_arith_type=on  arith_type=Unsigned  force_bin_pt=on  bin_pt=0  block_type=reinterpret
      settings: infoedit="Changes signal type without altering the binary representation.   You can changed the signal between signed and unsigned, and relocate the binary point.<br><br>Hardware notes: In hardware this block costs nothing.<br><br>Example:  Suppose the input is 6 bits wide, signed, with 2 fractional bits, and the output is forced to unsigned with 0 fractional bits.  Then an input of -2.0 (1110.00 in binary 2's complement) becomes an output of 56 (111000 in binary)."
  slice1 : xbsIndex_r4/Slice  nbits=8  boolean_output=off  mode=Upper Bit Location + Width  bit1=-24  base1=MSB of Input  bit0=0  base0=LSB of Input  dbl_ovrd=off  block_type=slice
  slice2 : xbsIndex_r4/Slice  nbits=8  boolean_output=off  mode=Upper Bit Location + Width  bit1=-16  base1=MSB of Input  bit0=0  base0=LSB of Input  dbl_ovrd=off  block_type=slice
  slice3 : xbsIndex_r4/Slice  nbits=8  boolean_output=off  mode=Upper Bit Location + Width  bit1=-8  base1=MSB of Input  bit0=0  base0=LSB of Input  dbl_ovrd=off  block_type=slice
  slice4 : xbsIndex_r4/Slice  nbits=8  boolean_output=off  mode=Upper Bit Location + Width  bit1=0  base1=MSB of Input  bit0=0  base0=LSB of Input  dbl_ovrd=off  block_type=slice
  msb_out4 : Outport
  out3 : Outport  Port=2
  out2 : Outport  Port=3
  lsb_out1 : Outport  Port=4
  wires:
  bus_in/1 -> slice4/1, slice3/1, slice2/1, slice1/1
  slice4/1 -> reinterpret4/1
  reinterpret4/1 -> msb_out4/1
  slice3/1 -> reinterpret3/1
  reinterpret3/1 -> out3/1
  slice2/1 -> reinterpret2/1
  reinterpret2/1 -> out2/1
  slice1/1 -> reinterpret1/1
  reinterpret1/1 -> lsb_out1/1

== system vegas01_4tap_1024ch_220_r7b/sg/bus_expand1 ==
  bus_in : Inport
  slice1 : xbsIndex_r4/Slice  nbits=1  boolean_output=on  mode=Upper Bit Location + Width  bit1=-7  base1=MSB of Input  bit0=0  base0=LSB of Input  dbl_ovrd=off  block_type=slice
  slice2 : xbsIndex_r4/Slice  nbits=1  boolean_output=on  mode=Upper Bit Location + Width  bit1=-6  base1=MSB of Input  bit0=0  base0=LSB of Input  dbl_ovrd=off  block_type=slice
  slice3 : xbsIndex_r4/Slice  nbits=1  boolean_output=on  mode=Upper Bit Location + Width  bit1=-5  base1=MSB of Input  bit0=0  base0=LSB of Input  dbl_ovrd=off  block_type=slice
  slice4 : xbsIndex_r4/Slice  nbits=1  boolean_output=on  mode=Upper Bit Location + Width  bit1=-4  base1=MSB of Input  bit0=0  base0=LSB of Input  dbl_ovrd=off  block_type=slice
  slice5 : xbsIndex_r4/Slice  nbits=1  boolean_output=on  mode=Upper Bit Location + Width  bit1=-3  base1=MSB of Input  bit0=0  base0=LSB of Input  dbl_ovrd=off  block_type=slice
  slice6 : xbsIndex_r4/Slice  nbits=1  boolean_output=on  mode=Upper Bit Location + Width  bit1=-2  base1=MSB of Input  bit0=0  base0=LSB of Input  dbl_ovrd=off  block_type=slice
  slice7 : xbsIndex_r4/Slice  nbits=1  boolean_output=on  mode=Upper Bit Location + Width  bit1=-1  base1=MSB of Input  bit0=0  base0=LSB of Input  dbl_ovrd=off  block_type=slice
  slice8 : xbsIndex_r4/Slice  nbits=1  boolean_output=on  mode=Upper Bit Location + Width  bit1=0  base1=MSB of Input  bit0=0  base0=LSB of Input  dbl_ovrd=off  block_type=slice
  msb_out8 : Outport
  out7 : Outport  Port=2
  out6 : Outport  Port=3
  out5 : Outport  Port=4
  out4 : Outport  Port=5
  out3 : Outport  Port=6
  out2 : Outport  Port=7
  lsb_out1 : Outport  Port=8
  wires:
  bus_in/1 -> slice8/1, slice7/1, slice6/1, slice5/1, slice4/1, slice3/1, slice2/1, slice1/1
  slice8/1 -> msb_out8/1
  slice7/1 -> out7/1
  slice6/1 -> out6/1
  slice5/1 -> out5/1
  slice4/1 -> out4/1
  slice3/1 -> out3/1
  slice2/1 -> out2/1
  slice1/1 -> lsb_out1/1

== system vegas01_4tap_1024ch_220_r7b/speader/bus_create ==
  in1 : Inport
  in2 : Inport  Port=2
  in3 : Inport  Port=3
  in4 : Inport  Port=4
  in5 : Inport  Port=5
  in6 : Inport  Port=6
  in7 : Inport  Port=7
  in8 : Inport  Port=8
  in9 : Inport  Port=9
  in10 : Inport  Port=10
  in11 : Inport  Port=11
  in12 : Inport  Port=12
  in13 : Inport  Port=13
  concatenate : xbsIndex_r4/Concat  num_inputs=13  dbl_ovrd=off  block_type=concat
  reinterpret{1..13} (13 alike) : xbsIndex_r4/Reinterpret  force_arith_type=on  arith_type=Unsigned  force_bin_pt=on  bin_pt=0  block_type=reinterpret
      reinterpret1 settings: infoedit="Changes signal type without altering the binary representation.   You can changed the signal between signed and unsigned, and relocate the binary point.<br><br>Hardware notes: In hardware this block costs nothing.<br><br>Example:  Suppose the input is 6 bits wide, signed, with 2 fractional bits, and the output is forced to unsigned with 0 fractional bits.  Then an input of -2.0 (1110.00 in binary 2's complement) becomes an output of 56 (111000 in binary)."
      reinterpret2 settings: infoedit="Changes signal type without altering the binary representation.   You can changed the signal between signed and unsigned, and relocate the binary point.<br><br>Hardware notes: In hardware this block costs nothing.<br><br>Example:  Suppose the input is 6 bits wide, signed, with 2 fractional bits, and the output is forced to unsigned with 0 fractional bits.  Then an input of -2.0 (1110.00 in binary 2's complement) becomes an output of 56 (111000 in binary)."
  bus_out : Outport
  wires:
  concatenate/1 -> bus_out/1
  in1/1 -> reinterpret1/1
  reinterpret1/1 -> concatenate/1
  in2/1 -> reinterpret2/1
  reinterpret2/1 -> concatenate/2
  in3/1 -> reinterpret3/1
  reinterpret3/1 -> concatenate/3
  in4/1 -> reinterpret4/1
  reinterpret4/1 -> concatenate/4
  in5/1 -> reinterpret5/1
  reinterpret5/1 -> concatenate/5
  in6/1 -> reinterpret6/1
  reinterpret6/1 -> concatenate/6
  in7/1 -> reinterpret7/1
  reinterpret7/1 -> concatenate/7
  in8/1 -> reinterpret8/1
  reinterpret8/1 -> concatenate/8
  in9/1 -> reinterpret9/1
  reinterpret9/1 -> concatenate/9
  in10/1 -> reinterpret10/1
  reinterpret10/1 -> concatenate/10
  in11/1 -> reinterpret11/1
  reinterpret11/1 -> concatenate/11
  in12/1 -> reinterpret12/1
  reinterpret12/1 -> concatenate/12
  in13/1 -> reinterpret13/1
  reinterpret13/1 -> concatenate/13
